simulink model "HDVSim_FCEV_Truck"  (R2019b) (continued)
== system HDVSim_FCEV_Truck/ECU == (continued)
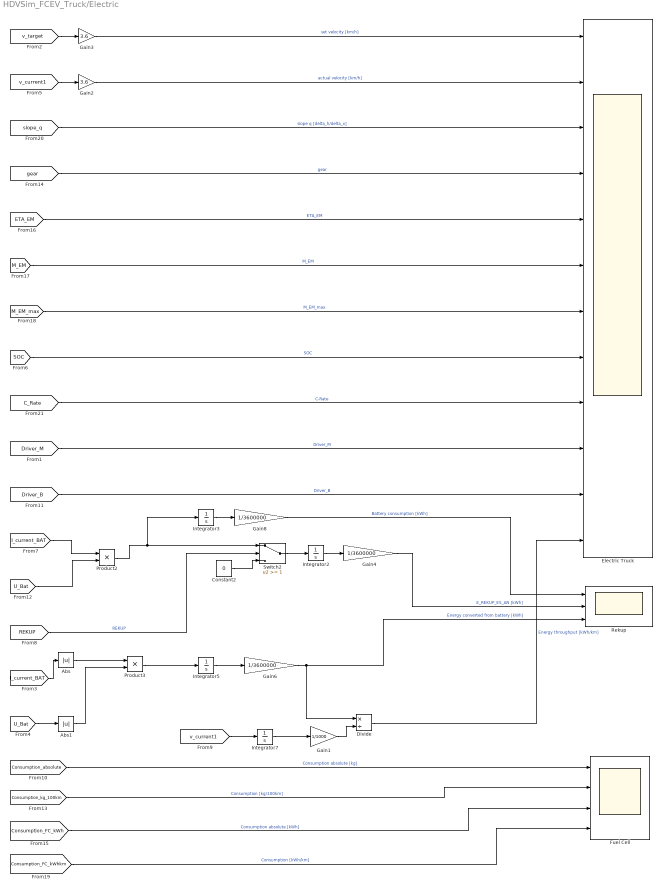
== system HDVSim_FCEV_Truck/Electric ==
  Abs : Abs
  Abs1 : Abs
  Constant2 : Constant  Value=0
  Divide : Product  Inputs=*/
  Electric Truck : Scope  NumInputPorts=12
  Fuel Cell : Scope  NumInputPorts=4
  Gain1 : Gain  Gain=1/1000
  Gain2 : Gain  Gain=3.6
  Gain3 : Gain  Gain=3.6
  Gain4 : Gain  Gain=1/3600000
  Gain6 : Gain  Gain=1/3600000
  Gain8 : Gain  Gain=1/3600000
  Integrator2 : Integrator
  Integrator3 : Integrator
  Integrator5 : Integrator
  Integrator7 : Integrator  InitialCondition=0.00000001
  Product2 : Product
  Product3 : Product
  Rekup : Scope  NumInputPorts=3
  Switch2 : Switch  Threshold=1
  wires:
  [v_target] -> Gain3/1
  Gain3/1 -> Electric Truck/1  "set velocity [km/h]"
  [v_current1] -> Gain2/1
  Gain2/1 -> Electric Truck/2  "actual velocity [km/h]"
  Gain4/1 -> Rekup/2  "E_REKUP_ES_AN [kWh]"
  Constant2/1 -> Switch2/3
  Integrator2/1 -> Gain4/1
  Switch2/1 -> Integrator2/1
  [REKUP] -> Switch2/2  "REKUP"
  [U_Bat] -> Product2/2
  [I_current_BAT] -> Product2/1
  [gear] -> Electric Truck/4  "gear"
  [ETA_EM] -> Electric Truck/5  "ETA_EM"
  [M_EM] -> Electric Truck/6  "M_EM"
  [M_EM_max] -> Electric Truck/7  "M_EM_max"
  [SOC] -> Electric Truck/8  "SOC"
  Product2/1 -> Integrator3/1, Switch2/1
  Gain8/1 -> Rekup/1  "Battery consumption [kWh]"
  Integrator3/1 -> Gain8/1
  [U_Bat] -> Abs1/1
  [I_current_BAT] -> Abs/1
  Product3/1 -> Integrator5/1
  Integrator5/1 -> Gain6/1
  Abs/1 -> Product3/1
  Abs1/1 -> Product3/2
  [slope_q] -> Electric Truck/3  "slope q [delta_h/delta_x]"
  [C_Rate] -> Electric Truck/9  "C-Rate"
  [Driver_M] -> Electric Truck/10  "Driver_M"
  [Driver_B] -> Electric Truck/11  "Driver_B"
  Gain6/1 -> Divide/1, Rekup/3  "Energy converted from battery [kWh]"
  Divide/1 -> Electric Truck/12  "Energy throughput [kWh/km]"
  [v_current1] -> Integrator7/1
  Integrator7/1 -> Gain1/1
  Gain1/1 -> Divide/2
  [Consumption_absolute] -> Fuel Cell/1  "Consumption absolute [kg]"
  [Consumption_kg_100km] -> Fuel Cell/2  "Consumption [kg/100km]"
  [Consumption_FC_kWh] -> Fuel Cell/3  "Consumption absolute [kWh]"
  [Consumption_FC_kWhkm] -> Fuel Cell/4  "Consumption [kWh/km]"

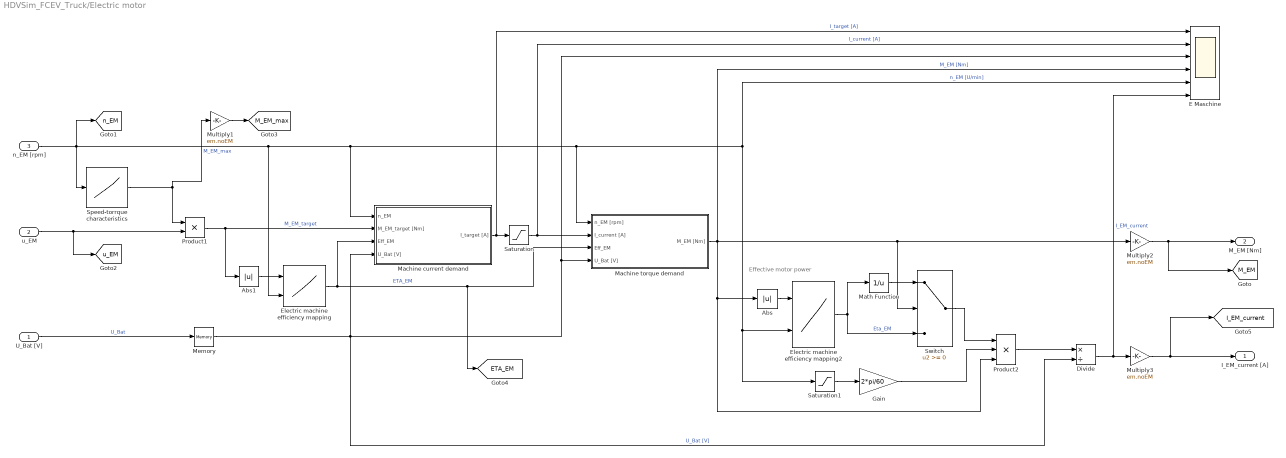
== system HDVSim_FCEV_Truck/Electric motor ==
  note: Effective motor power
  U_Bat [V] : Inport
  u_EM : Inport  Port=2
  n_EM [rpm] : Inport  Port=3
  Abs : Abs
  Abs1 : Abs
  Divide : Product  Inputs=*/
  E Maschine : Scope  NumInputPorts=6
  Electric machine efficiency mapping : Lookup_n-D  BreakpointsForDimension1=em.efficiency.torque  BreakpointsForDimension2=em. efficiency.speed  Table=em.efficiency.characteristic_map  RndMeth=Simplest  InputSameDT=on
  Electric machine efficiency mapping2 : Lookup_n-D  BreakpointsForDimension1=em.efficiency.torque  BreakpointsForDimension2=em. efficiency.speed  BeginIndexSearchUsingPreviousIndexResult=on  Table=em.efficiency.characteristic_map  ExtrapMethod=Clip  UseLastTableValue=on  RndMeth=Simplest  InputSameDT=on
  Gain : Gain  Gain=2*pi/60
  Machine current demand : Subsystem
  Machine torque demand : Subsystem
  Math Function : Math  Operator=reciprocal  SaturateOnIntegerOverflow=on
  Memory : Memory  InitialCondition=1.0943*Bat.Voltage
  Multiply1 : Gain  Gain=em.noEM
  Multiply2 : Gain  Gain=em.noEM
  Multiply3 : Gain  Gain=em.noEM
  Product1 : Product
  Product2 : Product  Inputs=3
  Saturation : Saturate  UpperLimit=400  LowerLimit=-400
  Saturation1 : Saturate  UpperLimit=em.n_max  LowerLimit=1
  Speed-torrque characteristics : Lookup_n-D  NumberOfTableDimensions=1  BreakpointsForDimension1=em.speed  BeginIndexSearchUsingPreviousIndexResult=on  Table=em.trq  ExtrapMethod=Clip  UseLastTableValue=on  RndMeth=Simplest  InputSameDT=on
  Switch : Switch
  I_EM_current [A] : Outport  VectorParamsAs1DForOutWhenUnconnected=off
  M_EM [Nm] : Outport  Port=2  VectorParamsAs1DForOutWhenUnconnected=off
  tag scope: ETA_EM=global, I_EM_current=global, M_EM=global, M_EM_max=global, n_EM=global, u_EM=global
  wires:
  Speed-torrque characteristics/1 -> Multiply1/1, Product1/1  "M_EM_max"
  u_EM/1 -> Product1/2, [u_EM]
  U_Bat [V]/1 -> Memory/1  "U_Bat"
  Machine current demand/1(I_target [A]) -> E Maschine/1, Saturation/1  "I_target [A]"
  Saturation/1 -> Machine torque demand/2(I_current [A]), E Maschine/2  "I_current [A]"
  Machine torque demand/1(M_EM [Nm]) -> Switch/2, Multiply2/1, E Maschine/4, Product2/3, Abs/1  "M_EM [Nm]"
  Gain/1 -> Product2/2
  Divide/1 -> E Maschine/6, Multiply3/1  "I_EM_current"
  Math Function/1 -> Switch/1
  Electric machine efficiency mapping2/1 -> Switch/3, Math Function/1  "Eta_EM"
  Switch/1 -> Product2/1
  Product2/1 -> Divide/1
  Abs/1 -> Electric machine efficiency mapping2/1
  Product1/1 -> Machine current demand/2(M_EM_target [Nm]), Abs1/1  "M_EM_target"
  n_EM [rpm]/1 -> Electric machine efficiency mapping/2, E Maschine/5, Saturation1/1, Electric machine efficiency mapping2/2, Machine torque demand/1(n_EM [rpm]), Machine current demand/1("n_EM "), [n_EM], Speed-torrque characteristics/1  "n_EM [U/min]"
  Abs1/1 -> Electric machine efficiency mapping/1
  Electric machine efficiency mapping/1 -> Machine current demand/3(Eff_EM), [ETA_EM], Machine torque demand/3(Eff_EM)  "ETA_EM"
  Saturation1/1 -> Gain/1
  Memory/1 -> Machine torque demand/4(U_Bat [V]), E Maschine/3, Divide/2, Machine current demand/4(U_Bat [V])  "U_Bat [V]"
  Multiply1/1 -> [M_EM_max]
  Multiply3/1 -> [I_EM_current], I_EM_current [A]/1
  Multiply2/1 -> [M_EM], M_EM [Nm]/1

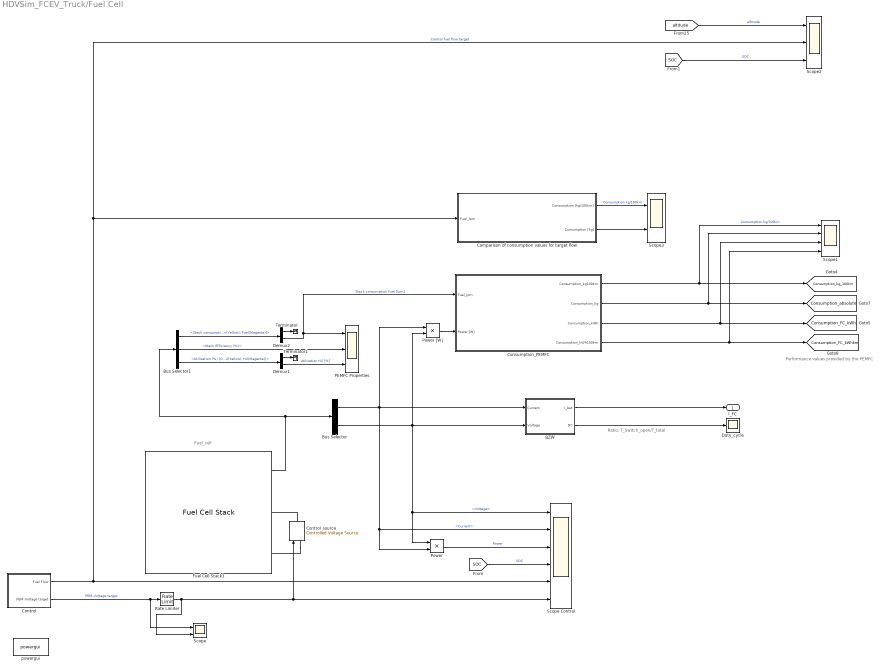
== system HDVSim_FCEV_Truck/Fuel Cell ==
  note: Fuel_cell
  note: Ratio: T_Switch_open/T_total
  note: Performance values provided by the PEMFC
  BZW : Subsystem
  Bus Selector : BusSelector  OutputSignals=Current,Voltage
  Bus Selector1 : BusSelector  OutputSignals=Stack consumption (lpm) [Air(Yellow); Fuel(Magenta)],Stack Efficiency (%),Utilization (%) [O2(Yellow); H2(Magenta)]
  Comparison of consumption values for target flow : Subsystem
  Consumption_PEMFC : Subsystem
  Control : Subsystem
  "Control_source " : powerlib/Electrical Sources/Controlled Voltage Source  Initialize=on  Source_Type=AC  Amplitude=0  Phase=0  Frequency=0  Measurements=None
  Demux1 : Demux  Outputs=2
  Demux2 : Demux  Outputs=2
  Duty_cycle : Scope
  Fuel Cell Stack1 : electricdrivelib/Extra Sources/Fuel Cell Stack  PresetModel=No (User-Defined)  FcType=0  Detailed=Detailed  Eoc=FuelCell.NernstVoltage  NomVI=FuelCell.OperatingPoint  EndVI=FuelCell.MaximumPoint  Nc=FuelCell.NoCells  n=FuelCell.Eta*0.999  TOp=FuelCell.OperatingTemp  AirFr=FuelCell.AirFlow  SuppPress=FuelCell.Pressure  Comp=FuelCell.Composition  SignX=off  SignY=off  FlowRateH2=off  FlowRateAir=off  SystemTemp=off  SystemPH2=off  SystemPAir=off  FCDyn=off  FC_tau=10  Peak_O2=80  V_Under=10
      settings: SourceProductBaseCode=PS
  PEMFC Properties : Scope  NumInputPorts=3
  Power : Product
  Power [W] : Product
  Rate Limiter : RateLimiter  RisingSlewLimit=100  FallingSlewLimit=-100  SampleTimeMode=inherited
  Scope : Scope  NumInputPorts=2
  Scope Control : Scope  NumInputPorts=6
  Scope1 : Scope  NumInputPorts=4
  Scope2 : Scope  NumInputPorts=3
  Scope3 : Scope  NumInputPorts=2
  Terminator : Terminator
  Terminator1 : Terminator
  powergui : powerlib/powergui  Priority=1  SimulationMode=Continuous  SampleTime=sample_time  frequency=60  Iterations=50  frequencyindice=0  Pbase=100e6  ErrMax=1e-4  UnitsV=kV  UnitsW=MW  buscounter=0  FunctionMessages=off  echomessages=off  EnableUseOfTLC=off  CurrentSourceSwitches=off  DisableSnubberDevices=off  DisableRonSwitches=off  DisableVfSwitches=off  DisplayEquations=off  AutomaticDiscreteSolvers=off  SolverType=Tustin/Backward Euler (TBE)  Interpol=off  ExternalGateDelay=off  methode=off  Ts=100  x0status=blocks  SPID=on  SwTol=0  HookPort=off  NonlinearTolerance=1e-4  nMaxIteration=100  ContinueOnMaxIteration=off  RestoreLinks=warning  ResistiveCurrentMeasurement=off  Frange=[0:2:500]  Ylog=off  Xlog=on  ShowGrid=off  save=off  variable=ZData  structure=0  ZoomFFT=off  StartTime=0.0  cycles=1  DisplayStyle=1  fundamental=60  FreqAxis=off  MaxFrequency=1000  frequencyindicesteady=1  RmsSteady=1  display=off
  I_FC : Outport  VectorParamsAs1DForOutWhenUnconnected=off
  tag scope: Consumption_FC_kWh=global, Consumption_FC_kWhkm=global, Consumption_absolute=global, Consumption_kg_100km=global
  wires:
  BZW/1(I_bat) -> I_FC/1
  BZW/2(DC) -> Duty_cycle/1
  Fuel Cell Stack1/1 -> Bus Selector/1, Bus Selector1/1
  Bus Selector/1 -> Power [W]/1, BZW/1(Current), Scope Control/2, Power/2  "<Current>"
  Bus Selector/2 -> Power [W]/2, Power/1, Scope Control/1, BZW/2(Voltage)  "<Voltage>"
  Control/2(PEM-Voltage target) -> Scope/1, Rate Limiter/1  "PEM-Voltage target"
  [SOC] -> Scope Control/4  "SOC"
  Power/1 -> Scope Control/3  "Power"
  Control/1(Fuel Flow) -> Scope2/2, Comparison of consumption values for target flow/1(Fuel_lpm), Scope Control/5  "Control fuel flow target"
  Consumption_PEMFC/1(Consumption_kg100km) -> [Consumption_kg_100km], Scope1/1  "Consumption kg/100km"
  Consumption_PEMFC/4(Consumption_kWh100km) -> Scope1/4, [Consumption_FC_kWhkm]
  Consumption_PEMFC/2(Consumption_kg) -> [Consumption_absolute], Scope1/2
  Consumption_PEMFC/3(Consumption_kWh) -> [Consumption_FC_kWh], Scope1/3
  Bus Selector1/2 -> PEMFC Properties/2  "<Stack Efficiency (%)>"
  Bus Selector1/1 -> Demux2/1  "<Stack consumption (lpm) [Air(Yellow); Fuel(Magenta)]>"
  Demux2/2 -> Consumption_PEMFC/1(Fuel_lpm), PEMFC Properties/1  "Stack consumption Fuel [lpm]"
  Demux2/1 -> Terminator/1
  Demux1/1 -> Terminator1/1
  Bus Selector1/3 -> Demux1/1  "<Utilization (%) [O2(Yellow); H2(Magenta)]>"
  Demux1/2 -> PEMFC Properties/3  "Utilization H2 [%]"
  Power [W]/1 -> Consumption_PEMFC/2(Power [W])
  [SOC] -> Scope2/3  "SOC"
  [altitude] -> Scope2/1  "altitude"
  Comparison of consumption values for target flow/1(Consumption [kg/100km]) -> Scope3/1  "Consumption kg/100km"
  Comparison of consumption values for target flow/2(Consumption [kg]) -> Scope3/2
  Rate Limiter/1 -> Scope/2, "Control_source "/1, Scope Control/6
  connections:
  "Control_source "/LConn1 -- Fuel Cell Stack1/RConn2
  "Control_source "/RConn1 -- Fuel Cell Stack1/RConn1

== system HDVSim_FCEV_Truck/Hybrid ==
  note: Battery parameters
  note: Battery energy
  note: Regenerative braking energy
  Abs : Abs
  Abs1 : Abs
  Constant1 : Constant  Value=0
  Display1 : Display
  Display5 : Display
  Display6 : Display
  Gain1 : Gain  Gain=1/3600000
  Gain6 : Gain  Gain=1/3600000
  Gain7 : Gain  Gain=1/3600000
  Integrator1 : Integrator
  Integrator5 : Integrator
  Integrator6 : Integrator
  Out_Bat : Scope  NumInputPorts=4
  Product1 : Product
  Product3 : Product
  Scope1 : Scope
  Scope3 : Scope
  Scope7 : Scope  NumInputPorts=2
  Switch1 : Switch  Threshold=1
  wires:
  Product1/1 -> Switch1/1, Integrator6/1
  Gain1/1 -> Scope7/1, Display1/1  "E_REKUP_ES_AN [kWh]"
  Constant1/1 -> Switch1/3
  Integrator1/1 -> Gain1/1
  Switch1/1 -> Integrator1/1
  [REKUP] -> Scope7/2, Switch1/2  "REKUP"
  [U_Bat] -> Product1/2
  [I_current_BAT] -> Product1/1
  [SOC] -> Out_Bat/1
  Gain7/1 -> Display6/1, Scope1/1
  Integrator6/1 -> Gain7/1
  [U_Bat] -> Abs1/1
  [I_current_BAT] -> Abs/1
  Product3/1 -> Integrator5/1
  Gain6/1 -> Display5/1, Scope3/1
  Integrator5/1 -> Gain6/1
  Abs/1 -> Product3/1
  Abs1/1 -> Product3/2
  [s_current1] -> Out_Bat/2
  [C_Rate] -> Out_Bat/3
  [I_EM_current] -> Out_Bat/4

== system HDVSim_FCEV_Truck/Power electronics and battery ==
  note: Total current demand
  note: Battery power and current demand
  I_PEM : Inport
  I_EM_current [A] : Inport  Port=2
  Battery1 : electricdrivelib/Extra Sources/Battery  BatType=Lithium-Ion  ShowTempParam=off  ThermalPreset=no  ShowAgeParam=off  NomV=Bat.Voltage  NomQ=Bat.Useable_capacity / Bat.Useable_range  SOC=(Bat.SOC_start *Bat.Useable_range+(0.5-Bat.Useable_range/2))* 100  Batt_Tr=30  PresetModel=off  MaxQ=Bat.Useable_capacity / Bat.Useable_range *8077/7500  MinV=487.5  FullV=Bat.Voltage * 1.178  Dis_rate=0.2 * Bat.Useable_capacity / Bat.Useable_range  R=0.01 * Bat.Voltage / (Bat.Useable_capacity / Bat.Useable_range)  Normal_OP=Bat.Useable_capacity / Bat.Useable_range * 0.962  expZone="[Bat.Voltage*4339/4000     Bat.Useable_capacity / Bat.Useable_range/5]"  current=[5 25 50 250]  Units=Ampere-hour  Tci=20  NomT=20  T2=-30  MaxQ2=4.8  FullV2=7.1  Normal_OP2=5.72  expZone2="[6.58  1]"  Rca=0.6  ti=1000  Dp=0  Neq0=0  Tsim=1e6  Ta1=25  Qeol=5.4*0.9  Reol=0.013333*1.2  Char_age=[2.3478, 3]  Dis_age=[2.3478, 10]  N1=1500  N2=10500  N3=1000  N4=1400  Ta2=45  N5=950
  Bus Selector : BusSelector  OutputSignals=SOC (%),Voltage (V)
  Controlled Current Source : powerlib/Electrical Sources/Controlled Current Source  Initialize=on  Source_Type=DC  Amplitude=0  Phase=0  Frequency=0  Measurements=None
  Conversion of SOC : Subsystem
  Divide : Product  Inputs=*/
  Divide1 : Product  Inputs=**
  Gain : Gain  Gain=-1
  Gain1 : Gain  Gain=1/100
  Gain2 : Gain  Gain=1/(Bat.Useable_capacity / Bat.Useable_range)
  Gain3 : Gain  Gain=1/1000
  I_EM : Display
  I_FC : Display
  I_NV : Display
  Memory : Memory
  P_aux [W] : Constant  Value=auxiliary_consumers.power
  Power electronics : Subsystem
  Scope5 : Scope
  Sum : Sum  Inputs=-++
  powergui : powerlib/powergui  Priority=1  SimulationMode=Discrete  SampleTime=sample_time  frequency=60  Iterations=50  frequencyindice=0  Pbase=100e6  ErrMax=1e-4  UnitsV=kV  UnitsW=MW  buscounter=0  FunctionMessages=off  echomessages=off  EnableUseOfTLC=off  CurrentSourceSwitches=off  DisableSnubberDevices=off  DisableRonSwitches=off  DisableVfSwitches=off  DisplayEquations=off  AutomaticDiscreteSolvers=off  SolverType=Tustin/Backward Euler (TBE)  Interpol=off  ExternalGateDelay=off  methode=off  Ts=100  x0status=blocks  SPID=on  SwTol=0  HookPort=off  NonlinearTolerance=1e-4  nMaxIteration=100  ContinueOnMaxIteration=off  RestoreLinks=warning  ResistiveCurrentMeasurement=off  Frange=[0:2:500]  Ylog=off  Xlog=on  ShowGrid=off  save=off  variable=ZData  structure=0  ZoomFFT=off  StartTime=0.0  cycles=1  DisplayStyle=1  fundamental=60  FreqAxis=off  MaxFrequency=1000  frequencyindicesteady=1  RmsSteady=1  display=off
  SOC : Outport  VectorParamsAs1DForOutWhenUnconnected=off
  U_Bat [V] : Outport  Port=2  VectorParamsAs1DForOutWhenUnconnected=off
  tag scope: C_Rate=global, I_current_BAT=global, SOC=global, U_Bat=global
  wires:
  Battery1/1 -> Bus Selector/1
  Bus Selector/1 -> Gain1/1  "<SOC (%)>"
  Bus Selector/2 -> [U_Bat], U_Bat [V]/1  "<Voltage (V)>"
  Gain1/1 -> Conversion of SOC/1(SOC_absolut)
  Memory/1 -> Gain/1
  Gain/1 -> Power electronics/1(I_PE_current [A])
  Gain2/1 -> [C_Rate]  "C Rate"
  [U_Bat] -> Divide/2
  P_aux [W]/1 -> Divide/1
  Divide/1 -> Sum/2, I_NV/1
  Sum/1 -> Memory/1
  I_EM_current [A]/1 -> Sum/3, I_EM/1
  Conversion of SOC/1(SOC_relative) -> SOC/1, [SOC]
  Gain3/1 -> Scope5/1
  [U_Bat] -> Divide1/1
  Power electronics/1(I_BAT_current [A]) -> [I_current_BAT], Gain2/1, Controlled Current Source/1, Divide1/2
  Divide1/1 -> Gain3/1
  I_PEM/1 -> I_FC/1, Sum/1
  connections:
  Controlled Current Source/LConn1 -- Battery1/LConn2
  Controlled Current Source/RConn1 -- Battery1/LConn1

== system HDVSim_FCEV_Truck/Tires ==
  M_tire [Nm] : Inport
  v_current [m/s] : Inport  Port=2
  F=M/r : Product  Inputs=*/
  Tire radius : Constant  Value=tires.radius
  "phi. -> n[1/min]" : Gain  Gain=60/2/pi
  phi. = v/r : Product  Inputs=/*
  n_tire [rpm] : Outport  VectorParamsAs1DForOutWhenUnconnected=off
  F_x [N] : Outport  Port=2  VectorParamsAs1DForOutWhenUnconnected=off
  wires:
  phi. = v/r/1 -> "phi. -> n[1/min]"/1
  "phi. -> n[1/min]"/1 -> n_tire [rpm]/1
  Tire radius/1 -> phi. = v/r/1, F=M/r/2
  v_current [m/s]/1 -> phi. = v/r/2
  M_tire [Nm]/1 -> F=M/r/1
  F=M/r/1 -> F_x [N]/1

== system HDVSim_FCEV_Truck/Transmission ==
  note: Transmission torque multiplication through the different gears
  M_EM [Nm] : Inport
  n_Gearbox [rpm] : Inport  Port=2
  v_target [m/s] : Inport  Port=3
  delta_v : Inport  Port=4
  Compare To Constant : simulink/Logic and Bit Operations/Compare To Constant  relop=~=  const=1  OutDataTypeStr=boolean
  Determine when shifting occurs : Subsystem  TreatAsAtomicUnit=on
  Memory1 : Memory
  Number of gears : Constant  Value=transmission.z  VectorParams1D=off
  Saturation : Saturate  UpperLimit=em.n_max  LowerLimit=0
  Shifting rules : Stateflow chart  TreatAsAtomicUnit=on
  Steigung-> Winkel : Trigonometry  Operator=atan
  Torque flow through the transmission : Subsystem
  Transmission gears : Constant  Value=transmission.z  VectorParams1D=off
  ZKU : Subsystem
  init_gear : Constant  Value=transmission.init_gear
  n_EM [rpm] : Outport  VectorParamsAs1DForOutWhenUnconnected=off
  M_Gearbox [Nm] : Outport  Port=2  VectorParamsAs1DForOutWhenUnconnected=off
  tag scope: gear=global, i_gearbox=global, recommend_DOWN_now=global, recommend_UP_now=global
  wires:
  n_Gearbox [rpm]/1 -> Torque flow through the transmission/2(n_gearbox_out [1/min]), Determine when shifting occurs/3(n_Gear_out [1/min])
  Torque flow through the transmission/2(M_gearbox_out [Nm]) -> [M_Gear_out], M_Gearbox [Nm]/1
  Torque flow through the transmission/1(n_gearbox_in [1/min]) -> Saturation/1
  init_gear/1 -> Shifting rules/1(init_gear)
  Determine when shifting occurs/27(check_torque_reseve_2lower) -> Shifting rules/29(check_torque_reserve_2lower)
  Determine when shifting occurs/26(check_torque_reseve_2higher) -> Shifting rules/28(check_torque_reserve_2higher)
  Determine when shifting occurs/1(reccommend_down_2higher) -> Shifting rules/3(recommend_down_2higher)
  Determine when shifting occurs/2(reccommend_down_1higher) -> Shifting rules/4(recommend_down_1higher)
  Determine when shifting occurs/3(reccommend_down_now) -> Shifting rules/5(recommend_down_now), [recommend_DOWN_now]
  Determine when shifting occurs/4(reccommend_down_1lower) -> Shifting rules/6(recommend_down_1lower)
  Determine when shifting occurs/5(reccommend_down_2lower) -> Shifting rules/7(recommend_down_2llower)
  Determine when shifting occurs/6(reccommend_up_2higher) -> Shifting rules/8(recommend_up_2higher)
  Determine when shifting occurs/7(reccommend_up_1higher) -> Shifting rules/9(recommend_up_1higher)
  Determine when shifting occurs/8(reccommend_up_now) -> Shifting rules/10(recommend_up_now), [recommend_UP_now]
  Determine when shifting occurs/9(reccommend_up_1lower) -> Shifting rules/11(recommend_up_1lower)
  Determine when shifting occurs/10(reccommend_up_2lower) -> Shifting rules/12(recommend_up_2lower)
  Determine when shifting occurs/11(check_n_min_2higher) -> Shifting rules/13(check_n_min_2higher)
  Determine when shifting occurs/12(check_n_min_1higher) -> Shifting rules/14(check_n_min_1higher)
  Determine when shifting occurs/13(check_n_min_now) -> Shifting rules/15(check_n_min_now)
  Determine when shifting occurs/14(check_n_min_1lower) -> Shifting rules/16(check_n_min_1lower)
  Determine when shifting occurs/15(check_n_min_2lower) -> Shifting rules/17(check_n_min_2lower)
  Determine when shifting occurs/16(check_n_max_2higher) -> Shifting rules/18(check_n_max_2higher)
  Determine when shifting occurs/17(check_n_max_1higher) -> Shifting rules/19(check_n_max_1higher)
  Determine when shifting occurs/18(check_n_max_now) -> Shifting rules/20(check_n_max_now)
  Determine when shifting occurs/19(check_n_max_1lower) -> Shifting rules/21(check_n_max_1lower)
  Determine when shifting occurs/20(check_n_max_2lower) -> Shifting rules/22(check_n_max_2lower)
  Determine when shifting occurs/21(check_M_max_2higher) -> Shifting rules/23(check_M_max_2higher)  "check_M_max_2higher"
  Determine when shifting occurs/22(check_M_max_1higher) -> Shifting rules/24(check_M_max_1higher)  "check_M_max_1higher"
  Determine when shifting occurs/23(check_M_max_now) -> Shifting rules/25(check_M_max_now)  "check_M_max_now"
  Determine when shifting occurs/24(check_M_max_1lower) -> Shifting rules/26(check_M_max_1lower)  "check_M_max_1lower"
  Determine when shifting occurs/25(check_M_max_2lower) -> Shifting rules/27(check_M_max_2lower)  "check_M_max_2lower"
  Memory1/1 -> [gear], [Gear], ZKU/1(Gear), Torque flow through the transmission/3(Gear)
  M_EM [Nm]/1 -> Torque flow through the transmission/1(M_gearbox_in [Nm])
  Saturation/1 -> n_EM [rpm]/1
  Torque flow through the transmission/3(i_gearbox) -> [i_gearbox]
  delta_v/1 -> Determine when shifting occurs/4(delta_v)
  v_target [m/s]/1 -> Determine when shifting occurs/5(v_target [m/s])
  Number of gears/1 -> Shifting rules/2(z)
  Transmission gears/1 -> Compare To Constant/1
  Compare To Constant/1 -> Determine when shifting occurs/Enable
  [slope_q] -> Steigung-> Winkel/1
  Steigung-> Winkel/1 -> Determine when shifting occurs/6(alpha)
  Shifting rules/1(gear) -> [gear]
  [gear] -> Memory1/1
  [Gear] -> Determine when shifting occurs/1(Gear)
  [M_Gear_out] -> Determine when shifting occurs/2(M_Gear_out [Nm])

== system HDVSim_FCEV_Truck/Vehicle ==
  note: Net tractive force applied on the vehicle
  note: Vehicle acceleration
  F_x [N] : Inport
  u_KB : Inport  Port=2
  alpha [rad] : Inport  Port=3
  Add : Sum  Inputs=-+-
  Cargo weight : Constant  Value=vehicle.payload
  Driving resistances without acceleration : Subsystem
  F_brake_max : Constant  Value=vehicle.F_brake_max
  Integrator : Integrator
  Integrator1 : Integrator  InitialCondition=cycle.speed_init  LimitOutput=on  LowerSaturationLimit=0
  Product : Product  Inputs=3
  Product2 : Product  Inputs=*/  RndMeth=Zero
  Product3 : Product  Inputs=**  RndMeth=Zero
  Sum1 : Sum  Inputs=|++
  Sum2 : Sum  Inputs=|++
  Vehicle weight : Constant  Value=vehicle.mass
  brake_force [N] : Product  RndMeth=Zero
  v_current [m/s] : Outport  VectorParamsAs1DForOutWhenUnconnected=off
  s_current [m] : Outport  Port=2  VectorParamsAs1DForOutWhenUnconnected=off
  tag scope: Acceletation_drag=global, s_current1=global, v_current1=global
  wires:
  alpha [rad]/1 -> Driving resistances without acceleration/1(alpha [rad])
  Add/1 -> Product2/1
  Driving resistances without acceleration/1(F_W [N]) -> Add/3
  F_x [N]/1 -> Add/2  "F_x"
  F_brake_max/1 -> brake_force [N]/2
  Integrator/1 -> [s_current1], s_current [m]/1  "s_current"
  Cargo weight/1 -> Sum1/2, Sum2/2
  Sum1/1 -> Product2/2
  Vehicle weight/1 -> Sum2/1, Product3/2
  Product3/1 -> Sum1/1
  Integrator1/1 -> v_current [m/s]/1, Driving resistances without acceleration/2(v [m/s]), [v_current1], Integrator/1  "v_current"
  [lamda] -> Product/1, Product3/1
  u_KB/1 -> brake_force [N]/1
  brake_force [N]/1 -> Add/1  "F_brake"
  Product2/1 -> Product/3, Integrator1/1  "a"
  Product/1 -> [Acceletation_drag]
  Sum2/1 -> Product/2  "m_total"

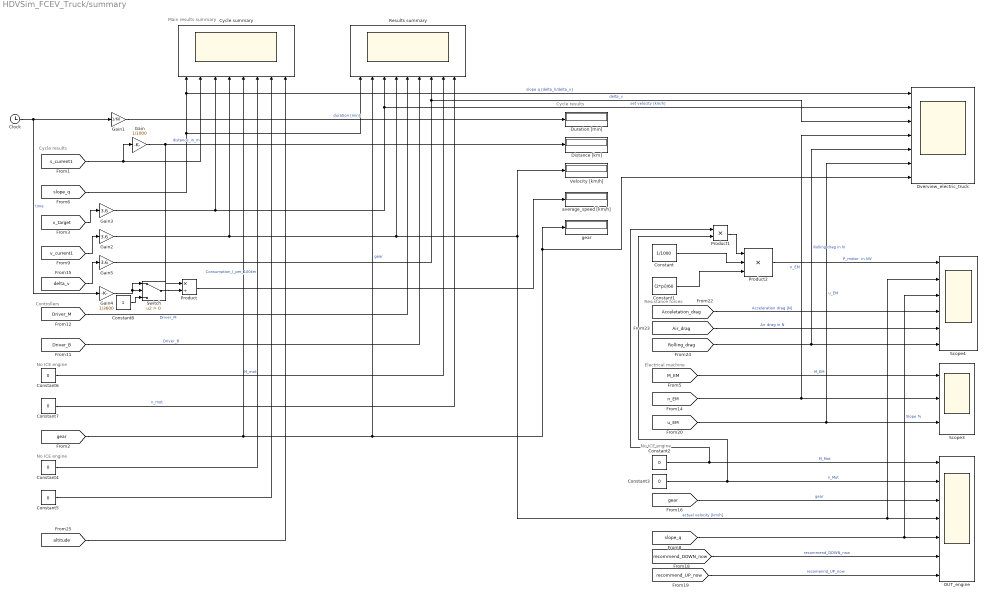
== system HDVSim_FCEV_Truck/summary ==
  note: No ICE engine
  note: No ICE engine
  note: Controllers
  note: Cycle results
  note: Main results summary
  note: Cycle results
  note: Resistance forces
  note: Electrical machine
  note: No ICE engine
  Clock : Clock
  Constant : Constant  Value=1/1000
  Constant1 : Constant  Value=(2*pi)/60
  Constant{2..7} (6 alike) : Constant  Value=0
  Constant8 : Constant
  Cycle summary : Scope  NumInputPorts=8
  Distance [km] : Display
  Duration [min] : Display
  Gain : Gain  Gain=1/1000
  Gain1 : Gain  Gain=1/60
  Gain2 : Gain  Gain=3.6
  Gain3 : Gain  Gain=3.6
  Gain4 : Gain  Gain=1/3600
  Gain5 : Gain  Gain=3.6
  OUT_engine : Scope  NumInputPorts=7
  Overview_electric_truck : Scope  NumInputPorts=7
  Product : Product  Inputs=*/  RndMeth=Zero
  Product1 : Product
  Product2 : Product  Inputs=3
  Results summary : Scope  NumInputPorts=9
  Scope3 : Scope  NumInputPorts=3
  Scope4 : Scope  NumInputPorts=6
  Switch : Switch  Criteria=u2 > Threshold
  Velocity [km/h] : Display
  average_speed [km/h] : Display
  gear : Display
  wires:
  [v_current1] -> Gain2/1
  [s_current1] -> Cycle summary/2, Gain/1  "distance_in_m"
  Gain/1 -> Distance [km]/1, Product/1
  Clock/1 -> Gain1/1, Gain4/1  "time"
  Gain1/1 -> Duration [min]/1  "duration [min]"
  Gain2/1 -> OUT_engine/4, Scope4/2, Velocity [km/h]/1, Results summary/4, Cycle summary/4  "actual velocity [km/h]"
  [gear] -> gear/1, Overview_electric_truck/7, Results summary/2, Cycle summary/5  "gear"
  [v_target] -> Gain3/1
  [slope_q] -> Results summary/1, Overview_electric_truck/1, Cycle summary/1  "slope q [delta_h/delta_x]"
  Constant4/1 -> Cycle summary/6  "Consumption_l_per_100km"
  Gain3/1 -> Results summary/3, Overview_electric_truck/2, Cycle summary/3  "set velocity [km/h]"
  Gain4/1 -> Switch/1, Switch/2
  Product/1 -> average_speed [km/h]/1
  Constant5/1 -> Cycle summary/7
  [Driver_M] -> Results summary/5  "Driver_M"
  [Driver_B] -> Results summary/6  "Driver_B"
  Constant6/1 -> Results summary/8  "M_mot"
  [delta_v] -> Gain5/1
  Constant2/1 -> OUT_engine/1, Product1/1  "M_Mot"
  Constant3/1 -> Product1/2, OUT_engine/2  "n_Mot"
  [gear] -> OUT_engine/3  "gear"
  [recommend_DOWN_now] -> OUT_engine/6  "recommend_DOWN_now"
  [recommend_UP_now] -> OUT_engine/7  "recomennd_UP_now"
  [slope_q] -> Scope4/3, OUT_engine/5  "Slope %"
  Gain5/1 -> Results summary/7, Overview_electric_truck/3  "delta_v"
  Constant7/1 -> Results summary/9  "n_mot"
  [M_EM] -> Scope3/1  "M_EM"
  [n_EM] -> Overview_electric_truck/4, Scope3/2  "n_EM"
  [u_EM] -> Overview_electric_truck/6, Scope3/3  "u_EM"
  Product1/1 -> Product2/1
  Constant/1 -> Product2/2
  Product2/1 -> Scope4/1  "P_motor  in kW"
  Constant1/1 -> Product2/3
  [Acceletation_drag] -> Scope4/4  "Acceleration drag [N]"
  [Air_drag] -> Scope4/5  "Air drag in N"
  [Rolling_drag] -> Overview_electric_truck/5, Scope4/6  "Rolling drag in N"
  Switch/1 -> Product/2
  Constant8/1 -> Switch/3
  [altitude] -> Cycle summary/8

== system HDVSim_FCEV_Truck/Active cruise control1/Braking from upper speed limit ==
  v_target [m/s] : Inport
  Add3 : Sum  Inputs=+-
  Constant1 : Constant  Value=90/3.6
  Constant10 : Constant  Value=prospective_cruise_control
  Constant6 : Constant  Value=v_PPC_delta
  Constant7 : Constant  Value=0
  Constant8 : Constant  Value=0
  Saturation Dynamic : simulink/Discontinuities/Saturation Dynamic  OutMin=[]  OutMax=[]  OutDataTypeStr=Inherit: Same as second input  OutDataType=fixdt(1, 16)  OutScaling=2^-10  DoSatur=off
  Switch3 : Switch  Criteria=u2 > Threshold
  Out1 : Outport  VectorParamsAs1DForOutWhenUnconnected=off
  wires:
  Switch3/1 -> Out1/1
  Constant6/1 -> Saturation Dynamic/2
  Constant1/1 -> Add3/1
  Constant7/1 -> Switch3/3
  Add3/1 -> Saturation Dynamic/1
  Constant8/1 -> Saturation Dynamic/3
  Constant10/1 -> Switch3/2
  v_target [m/s]/1 -> Add3/2
  Saturation Dynamic/1 -> Switch3/1
